simulink model "ol_simulation"  (R2023b)
settings: Solver=FixedStepAuto  StartTime=0.0  StopTime=10  FixedStep=0.05  RelTol=1e-3
model workspace (MAT data in the model file):
  Logfile_Data_Object : robotics.ros.BagSelection (value not decoded)
  Logfile_Path = '/Users/sprinkle/work/teaching/cs3891-2023F/src/docker/mytest.bag'
  Logfile_Playback_Duration = 200
  Logfile_Playback_Offset = 100
libraries: simulink x1
settings Model: Creator=sprinkle  LastModifiedBy=marystirlingbrown
settings SolverCC: AlgebraicLoopSolver=TrustRegion
settings OptimizationCC: EnhancedBackFolding=on  PassReuseOutputArgsAs=Individual arguments  ZeroExternalMemoryAtStartup=off  ZeroInternalMemoryAtStartup=off  MaxStackSize=64  OptimizeBlockOrder=speed  DifferentSizesBufferReuse=on  OptimizationCustomize=off  EfficientTunableParamExpr=on
settings DebuggingCC: ParameterTunabilityLossMsg=error  UnconnectedInputMsg=warning  UnconnectedOutputMsg=warning  UnconnectedLineMsg=warning
settings HardwareCC: ProdBitPerPointer=32  ProdBitPerSizeT=32  ProdBitPerPtrDiffT=32  ProdLargestAtomicFloat=None  ProdIntDivRoundTo=Undefined  ProdEndianess=Unspecified  ProdWordSize=32  ProdHWDeviceType=Generic->Unspecified (assume 32-bit Generic)
settings ModelReferenceCC: EnableRefExpFcnMdlSchedulingChecks=on
settings SFSimCC: SimTargetLang=C++
settings RTWCC: Description=Embedded Coder  SystemTargetFile=ert.tlc  HardwareBoard=Robot Operating System (ROS)  TLCOptions="-aInlineSetEventsForThisBaseRateFcn=TLC_FALSE -aSuppressMultiTaskScheduler=TLC_FALSE  -aRateBasedStepFcn=0"  TemplateMakefile=ert_default_tmf  Toolchain=Catkin  TargetLang=C++
settings CodeAppCC: ForceParamTrailComments=on  IgnoreCustomStorageClasses=off  OperatorAnnotations=on  SimulinkDataObjDesc=on  SFDataObjDesc=on  InsertBlockDesc=on
settings ERTTargetCC: SupportContinuousTime=on

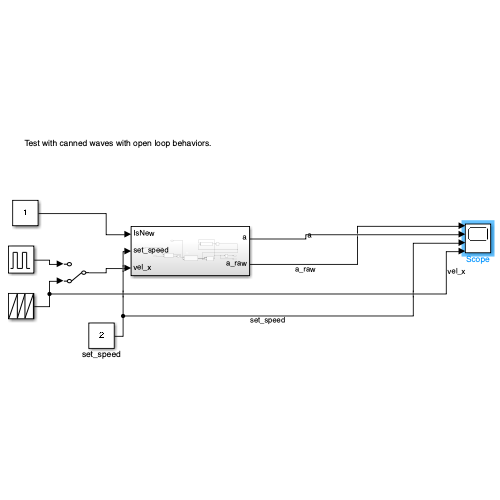
[thumbnail: Simulink's stored preview of the top-level diagram]
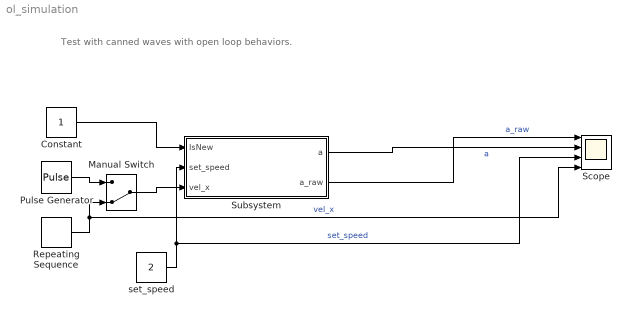
== system ol_simulation ==
  note: Test with canned waves with open loop behaviors.
  Constant : Constant
  Manual Switch : ManualSwitch  CurrentSetting=0
  Pulse Generator : DiscretePulseGenerator  PulseType=Time based  Amplitude=2  Period=10  PulseWidth=30
  Repeating Sequence : simulink/Sources/Repeating Sequence  rep_seq_t=[0 10]  rep_seq_y=[-2 2]
  Scope : Scope  NumInputPorts=4
  Subsystem : Subsystem
  set_speed : Constant  Value=2
  wires:
  Subsystem/1(a) -> Scope/2  "a"
  Subsystem/2(a_raw) -> Scope/1  "a_raw"
  Constant/1 -> Subsystem/1(IsNew)
  Manual Switch/1 -> Subsystem/3(vel_x)
  Pulse Generator/1 -> Manual Switch/1
  set_speed/1 -> Scope/3, Subsystem/2(set_speed)  "set_speed"
  Repeating Sequence/1 -> Scope/4, Manual Switch/2  "vel_x"

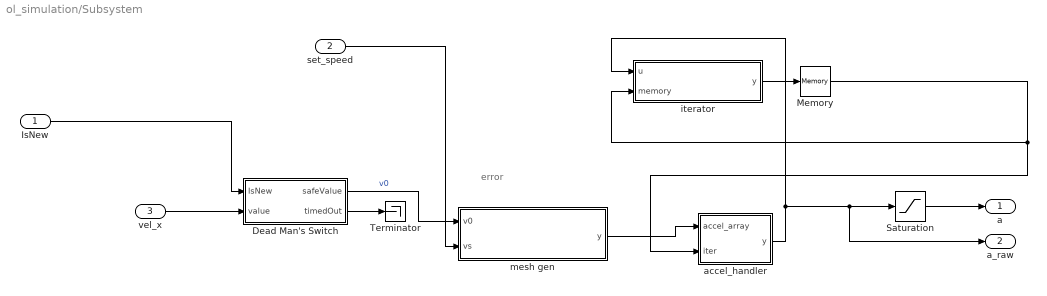
== system ol_simulation/Subsystem ==
  note: error
  IsNew : Inport
  set_speed : Inport  Port=2
  vel_x : Inport  Port=3
  Dead Man's Switch : Subsystem  TreatAsAtomicUnit=on  mask{stepSize=0.05, timeout=0.4}
      mask prompts: stepSize "Simulation step size"; timeout "Timeout (in seconds)"
      mask help: Interprets whether a new message has been recently received, and if so permits the data in 'value' to be passed through. Otherwise, 0 is outputted from 'safeValue'
      mask Help: Interprets whether a new message has been recently received, and if so permits the data in 'value' to be passed through. Otherwise, 0 is outputted from 'safeValue'
  Memory : Memory  InitialCondition=1
  Saturation : Saturate  UpperLimit=1.5  LowerLimit=-3
  Terminator : Terminator
  accel_handler : MATLAB Function  TreatAsAtomicUnit=on
  iterator : MATLAB Function  TreatAsAtomicUnit=on
  mesh gen : MATLAB Function  TreatAsAtomicUnit=on
  a : Outport
  a_raw : Outport  Port=2
  wires:
  IsNew/1 -> Dead Man's Switch/1(IsNew)
  Dead Man's Switch/2(timedOut) -> Terminator/1
  vel_x/1 -> Dead Man's Switch/2(value)
  Saturation/1 -> a/1
  Dead Man's Switch/1(safeValue) -> mesh gen/1(v0)  "v0"
  Memory/1 -> accel_handler/2(iter), iterator/2(memory)
  iterator/1(y) -> Memory/1
  mesh gen/1(y) -> accel_handler/1(accel_array)
  set_speed/1 -> mesh gen/2(vs)
  accel_handler/1(y) -> a_raw/1, Saturation/1, iterator/1(u)

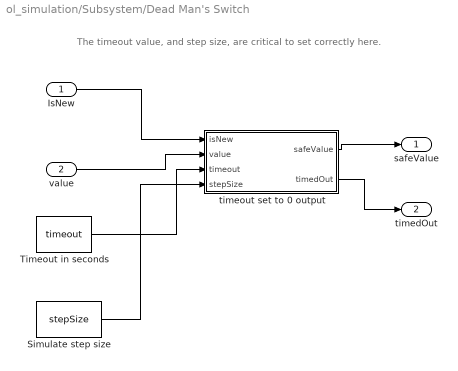
== system ol_simulation/Subsystem/Dead Man's Switch ==
  note: The timeout value, and step size, are critical to set correctly here.
  IsNew : Inport
  value : Inport  Port=2
  Simulate step size : Constant  Value=stepSize
  Timeout in seconds : Constant  Value=timeout
  timeout set to 0 output : MATLAB Function  TreatAsAtomicUnit=on
  safeValue : Outport  InitialOutput=[0]
  timedOut : Outport  Port=2  InitialOutput=[0]
  wires:
  IsNew/1 -> timeout set to 0 output/1(isNew)
  timeout set to 0 output/1(safeValue) -> safeValue/1
  value/1 -> timeout set to 0 output/2(value)
  Timeout in seconds/1 -> timeout set to 0 output/3(timeout)
  timeout set to 0 output/2(timedOut) -> timedOut/1
  Simulate step size/1 -> timeout set to 0 output/4(stepSize)

== matlab function ol_simulation/Subsystem/accel_handler ==
  data: accel_array input; y output; iter input
function y = fcn(accel_array, iter)

y = accel_array(iter)

== matlab function ol_simulation/Subsystem/Dead Man's Switch/timeout set to 0 output ==
  settings: updateMethod=DISCRETE
  data: isNew input; safeValue output; value input; timeout input; timedOut output; stepSize input
function [safeValue,timedOut] = fcn(isNew,value,timeout,stepSize)
%#codegen
persistent sinceLastMsg
timedOut=true;
if isempty(sinceLastMsg)
    sinceLastMsg=(timeout/stepSize)+1;
end

safeValue=0;
% if no new message
% after timeout, we output 0
if( isNew == true )
    sinceLastMsg = 0;
else
    sinceLastMsg = sinceLastMsg+1;
end

% Note: we require step size as an input here, and depend on our
% system being executed at a regular rate, or bad things will happen
if( sinceLastMsg < timeout/stepSize )
    timedOut = false;
end
    
if( timedOut == false )
    safeValue = value;
end

== matlab function ol_simulation/Subsystem/mesh gen ==
  settings: updateMethod=DISCRETE  sampleTime=0.1
  data: v0 input; y output; vs input
function y = fcn(v0, vs)

    time = 10;          % time gap
    midpoint = time / 2; % describes midpoint of time

    % mesh_data = zeros(1000);
    mesh_data = zeros(1, 1000);
    if vs > v0
        % accelerate
        accel_mid = (vs - v0) / 2;
        mesh_data = [accel_mid];
        y_val = accel_mid;
        % build first part of acceleration mesh
        for i = 1:(midpoint * 10)
            % scaling with time to ensure convergence
            y_val = y_val / ((time + 1) / time);
            mesh_data = [y_val, mesh_data];
        end
        % Reset variables, declare second half of mesh
        y_val = accel_mid;
        sec_mesh = [];
        set_tmp = vs - v0;
        % construct second half of acceleration mesh
        for i = 1:(midpoint * 10)
            % remove next smallest increment obtained from set speed
            set_tmp = set_tmp - mesh_data(i);
            sec_mesh = [set_tmp, sec_mesh];
            set_tmp = vs - v0;
        end
        % concatenate meshes to form one mesh with h = 0.1
        mesh_data = [mesh_data, sec_mesh];
        
        y = mesh_data;
    elseif vs < v0
        % decelerate
        decel_mid = (v0 - vs) / 2;
        mesh_data = decel_mid;
        y_val = decel_mid;
        % build second half of mesh
        for i = 1:(midpoint * 10)
            % scale with time
            y_val = y_val / ((time + 1) / time);
            mesh_data = [mesh_data, y_val];
        end
        % Reset variables, init first half of mesh
        y_val = decel_mid;
        sec_mesh = [];
        set_tmp = v0 - vs;
        mesh_data = fliplr(mesh_data); % reversed to obtain smallest values first
        % similar in construction to first
        for i = 1:(midpoint * 10)
            set_tmp = set_tmp - mesh_data(i);
            sec_mesh = [sec_mesh, set_tmp];
            set_tmp = v0-vs;
        end
        % Reversed such that it decays from midpoint again
        mesh_data = fliplr(mesh_data);
        % Concatenate meshes
        mesh_data = [sec_mesh, mesh_data];
        y = mesh_data;
    else
        y = mesh_data;
    end

    my_spline = spline(0:0.1:10, mesh_data);
    derivative_array = [];
    a = 0.0;
    for idx = 1:length(mesh_data)  % Use an index variable instead of "for v = mesh_data"
        derivative_array = [derivative_array, (ppval(my_spline, a+0.1) - ppval(my_spline, a))/0.1];
        a = a + 0.1;
    end
    accel_array = derivative_array;
    % return accel_array
    y = accel_array;
end

== matlab function ol_simulation/Subsystem/iterator ==
  settings: updateMethod=DISCRETE  sampleTime=0.1
  data: u input; y output; memory input
function y = fcn(u, memory)

iter = memory;



if u ~= 0 && iter < 100
    iter = iter + 1;
end


y = iter;
